simulink model "V2x_TC_TrafficLights_cs"  (Simulink 7.6)
settings: Solver=ode5  SolverMode=SingleTasking  StartTime=0.0  StopTime=38  FixedStep=1/100  RelTol=1e-3
PreLoadFcn:
  | check_NUTS_defined
  | 
  | assignin('base',['model_closed_' bdroot],false);addpath('../includes_V2x');
InitFcn:
  | PreScanExperimentInitFcn(bdroot)
StopFcn:
  | PreScanExperimentStopFcn(bdroot);
  | 
undefined symbols (not defined in the file or in its folder): acceleration, actorID, actorType, DiscIntNeverNeededParam, GPS_alt, GPS_lat, GPS_long, intmax, lightBarInUse, messageID, Newton, publicActorType, Raphson, rotZ, sigbuilder_block, sireneInUse, trafficLightPriority, turnAdvice, velocity, x_ini_VRML, y_ini_VRML, yawRate, z_ini_VRML
libraries: simulink x172, PreScanSimulinkLibrary x20, PreScanUsefulBlocks x11, simulink_extras x9, simulink_need_slupdate x1
(part 2 of 27)

== system V2x_TC_TrafficLights_cs/Antenna_Rx_1 ==
  Selector : Selector  IndexMode=Zero-based  IndexOptions=Starting index (dialog)  Indices=PortIndex  OutputSizes=PortWidth(1)
  U : Outport
  wires:
  Selector/1 -> U/1
  [SimCoreSensors] -> Selector/1

== system V2x_TC_TrafficLights_cs/Antenna_Rx_2 ==
  Selector : Selector  IndexMode=Zero-based  IndexOptions=Starting index (dialog)  Indices=PortIndex  OutputSizes=PortWidth(1)
  U : Outport
  wires:
  Selector/1 -> U/1
  [SimCoreSensors] -> Selector/1

== system V2x_TC_TrafficLights_cs/Antenna_Rx_3 ==
  Selector : Selector  IndexMode=Zero-based  IndexOptions=Starting index (dialog)  Indices=PortIndex  OutputSizes=PortWidth(1)
  U : Outport
  wires:
  Selector/1 -> U/1
  [SimCoreSensors] -> Selector/1

== system V2x_TC_TrafficLights_cs/Antenna_Rx_4 ==
  Selector : Selector  IndexMode=Zero-based  IndexOptions=Starting index (dialog)  Indices=PortIndex  OutputSizes=PortWidth(1)
  U : Outport
  wires:
  Selector/1 -> U/1
  [SimCoreSensors] -> Selector/1

== system V2x_TC_TrafficLights_cs/Antenna_Rx_5 ==
  Selector : Selector  IndexMode=Zero-based  IndexOptions=Starting index (dialog)  Indices=PortIndex  OutputSizes=PortWidth(1)
  U : Outport
  wires:
  Selector/1 -> U/1
  [SimCoreSensors] -> Selector/1

== system V2x_TC_TrafficLights_cs/Antenna_Tx_1 ==
  Y : Inport
  Assignment : Assignment  IndexMode=Zero-based  SampleTime=TimeStep  IndexOptions=Starting index (dialog)  Indices=PortIndex  OutputSizes=[1 1]
  Data Store Read : DataStoreRead  DataStoreName=SimCoreActuators  SampleTime=TimeStep
  Data Store Write : DataStoreWrite  DataStoreName=SimCoreActuators
  wires:
  Assignment/1 -> Data Store Write/1
  Data Store Read/1 -> Assignment/1
  Y/1 -> Assignment/2

== system V2x_TC_TrafficLights_cs/Antenna_Tx_10 ==
  Y : Inport
  Assignment : Assignment  IndexMode=Zero-based  SampleTime=TimeStep  IndexOptions=Starting index (dialog)  Indices=PortIndex  OutputSizes=[1 1]
  Data Store Read : DataStoreRead  DataStoreName=SimCoreActuators  SampleTime=TimeStep
  Data Store Write : DataStoreWrite  DataStoreName=SimCoreActuators
  wires:
  Assignment/1 -> Data Store Write/1
  Data Store Read/1 -> Assignment/1
  Y/1 -> Assignment/2

== system V2x_TC_TrafficLights_cs/Antenna_Tx_11 ==
  Y : Inport
  Assignment : Assignment  IndexMode=Zero-based  SampleTime=TimeStep  IndexOptions=Starting index (dialog)  Indices=PortIndex  OutputSizes=[1 1]
  Data Store Read : DataStoreRead  DataStoreName=SimCoreActuators  SampleTime=TimeStep
  Data Store Write : DataStoreWrite  DataStoreName=SimCoreActuators
  wires:
  Assignment/1 -> Data Store Write/1
  Data Store Read/1 -> Assignment/1
  Y/1 -> Assignment/2

== system V2x_TC_TrafficLights_cs/Antenna_Tx_12 ==
  Y : Inport
  Assignment : Assignment  IndexMode=Zero-based  SampleTime=TimeStep  IndexOptions=Starting index (dialog)  Indices=PortIndex  OutputSizes=[1 1]
  Data Store Read : DataStoreRead  DataStoreName=SimCoreActuators  SampleTime=TimeStep
  Data Store Write : DataStoreWrite  DataStoreName=SimCoreActuators
  wires:
  Assignment/1 -> Data Store Write/1
  Data Store Read/1 -> Assignment/1
  Y/1 -> Assignment/2

== system V2x_TC_TrafficLights_cs/Antenna_Tx_13 ==
  Y : Inport
  Assignment : Assignment  IndexMode=Zero-based  SampleTime=TimeStep  IndexOptions=Starting index (dialog)  Indices=PortIndex  OutputSizes=[1 1]
  Data Store Read : DataStoreRead  DataStoreName=SimCoreActuators  SampleTime=TimeStep
  Data Store Write : DataStoreWrite  DataStoreName=SimCoreActuators
  wires:
  Assignment/1 -> Data Store Write/1
  Data Store Read/1 -> Assignment/1
  Y/1 -> Assignment/2

== system V2x_TC_TrafficLights_cs/Antenna_Tx_14 ==
  Y : Inport
  Assignment : Assignment  IndexMode=Zero-based  SampleTime=TimeStep  IndexOptions=Starting index (dialog)  Indices=PortIndex  OutputSizes=[1 1]
  Data Store Read : DataStoreRead  DataStoreName=SimCoreActuators  SampleTime=TimeStep
  Data Store Write : DataStoreWrite  DataStoreName=SimCoreActuators
  wires:
  Assignment/1 -> Data Store Write/1
  Data Store Read/1 -> Assignment/1
  Y/1 -> Assignment/2

== system V2x_TC_TrafficLights_cs/Antenna_Tx_15 ==
  Y : Inport
  Assignment : Assignment  IndexMode=Zero-based  SampleTime=TimeStep  IndexOptions=Starting index (dialog)  Indices=PortIndex  OutputSizes=[1 1]
  Data Store Read : DataStoreRead  DataStoreName=SimCoreActuators  SampleTime=TimeStep
  Data Store Write : DataStoreWrite  DataStoreName=SimCoreActuators
  wires:
  Assignment/1 -> Data Store Write/1
  Data Store Read/1 -> Assignment/1
  Y/1 -> Assignment/2

== system V2x_TC_TrafficLights_cs/Antenna_Tx_16 ==
  Y : Inport
  Assignment : Assignment  IndexMode=Zero-based  SampleTime=TimeStep  IndexOptions=Starting index (dialog)  Indices=PortIndex  OutputSizes=[1 1]
  Data Store Read : DataStoreRead  DataStoreName=SimCoreActuators  SampleTime=TimeStep
  Data Store Write : DataStoreWrite  DataStoreName=SimCoreActuators
  wires:
  Assignment/1 -> Data Store Write/1
  Data Store Read/1 -> Assignment/1
  Y/1 -> Assignment/2

== system V2x_TC_TrafficLights_cs/Antenna_Tx_17 ==
  Y : Inport
  Assignment : Assignment  IndexMode=Zero-based  SampleTime=TimeStep  IndexOptions=Starting index (dialog)  Indices=PortIndex  OutputSizes=[1 1]
  Data Store Read : DataStoreRead  DataStoreName=SimCoreActuators  SampleTime=TimeStep
  Data Store Write : DataStoreWrite  DataStoreName=SimCoreActuators
  wires:
  Assignment/1 -> Data Store Write/1
  Data Store Read/1 -> Assignment/1
  Y/1 -> Assignment/2

== system V2x_TC_TrafficLights_cs/Antenna_Tx_18 ==
  Y : Inport
  Assignment : Assignment  IndexMode=Zero-based  SampleTime=TimeStep  IndexOptions=Starting index (dialog)  Indices=PortIndex  OutputSizes=[1 1]
  Data Store Read : DataStoreRead  DataStoreName=SimCoreActuators  SampleTime=TimeStep
  Data Store Write : DataStoreWrite  DataStoreName=SimCoreActuators
  wires:
  Assignment/1 -> Data Store Write/1
  Data Store Read/1 -> Assignment/1
  Y/1 -> Assignment/2

== system V2x_TC_TrafficLights_cs/Antenna_Tx_19 ==
  Y : Inport
  Assignment : Assignment  IndexMode=Zero-based  SampleTime=TimeStep  IndexOptions=Starting index (dialog)  Indices=PortIndex  OutputSizes=[1 1]
  Data Store Read : DataStoreRead  DataStoreName=SimCoreActuators  SampleTime=TimeStep
  Data Store Write : DataStoreWrite  DataStoreName=SimCoreActuators
  wires:
  Assignment/1 -> Data Store Write/1
  Data Store Read/1 -> Assignment/1
  Y/1 -> Assignment/2

== system V2x_TC_TrafficLights_cs/Antenna_Tx_2 ==
  Y : Inport
  Assignment : Assignment  IndexMode=Zero-based  SampleTime=TimeStep  IndexOptions=Starting index (dialog)  Indices=PortIndex  OutputSizes=[1 1]
  Data Store Read : DataStoreRead  DataStoreName=SimCoreActuators  SampleTime=TimeStep
  Data Store Write : DataStoreWrite  DataStoreName=SimCoreActuators
  wires:
  Assignment/1 -> Data Store Write/1
  Data Store Read/1 -> Assignment/1
  Y/1 -> Assignment/2

== system V2x_TC_TrafficLights_cs/Antenna_Tx_20 ==
  Y : Inport
  Assignment : Assignment  IndexMode=Zero-based  SampleTime=TimeStep  IndexOptions=Starting index (dialog)  Indices=PortIndex  OutputSizes=[1 1]
  Data Store Read : DataStoreRead  DataStoreName=SimCoreActuators  SampleTime=TimeStep
  Data Store Write : DataStoreWrite  DataStoreName=SimCoreActuators
  wires:
  Assignment/1 -> Data Store Write/1
  Data Store Read/1 -> Assignment/1
  Y/1 -> Assignment/2

== system V2x_TC_TrafficLights_cs/Antenna_Tx_21 ==
  Y : Inport
  Assignment : Assignment  IndexMode=Zero-based  SampleTime=TimeStep  IndexOptions=Starting index (dialog)  Indices=PortIndex  OutputSizes=[1 1]
  Data Store Read : DataStoreRead  DataStoreName=SimCoreActuators  SampleTime=TimeStep
  Data Store Write : DataStoreWrite  DataStoreName=SimCoreActuators
  wires:
  Assignment/1 -> Data Store Write/1
  Data Store Read/1 -> Assignment/1
  Y/1 -> Assignment/2

== system V2x_TC_TrafficLights_cs/Antenna_Tx_22 ==
  Y : Inport
  Assignment : Assignment  IndexMode=Zero-based  SampleTime=TimeStep  IndexOptions=Starting index (dialog)  Indices=PortIndex  OutputSizes=[1 1]
  Data Store Read : DataStoreRead  DataStoreName=SimCoreActuators  SampleTime=TimeStep
  Data Store Write : DataStoreWrite  DataStoreName=SimCoreActuators
  wires:
  Assignment/1 -> Data Store Write/1
  Data Store Read/1 -> Assignment/1
  Y/1 -> Assignment/2

== system V2x_TC_TrafficLights_cs/Antenna_Tx_23 ==
  Y : Inport
  Assignment : Assignment  IndexMode=Zero-based  SampleTime=TimeStep  IndexOptions=Starting index (dialog)  Indices=PortIndex  OutputSizes=[1 1]
  Data Store Read : DataStoreRead  DataStoreName=SimCoreActuators  SampleTime=TimeStep
  Data Store Write : DataStoreWrite  DataStoreName=SimCoreActuators
  wires:
  Assignment/1 -> Data Store Write/1
  Data Store Read/1 -> Assignment/1
  Y/1 -> Assignment/2

== system V2x_TC_TrafficLights_cs/Antenna_Tx_3 ==
  Y : Inport
  Assignment : Assignment  IndexMode=Zero-based  SampleTime=TimeStep  IndexOptions=Starting index (dialog)  Indices=PortIndex  OutputSizes=[1 1]
  Data Store Read : DataStoreRead  DataStoreName=SimCoreActuators  SampleTime=TimeStep
  Data Store Write : DataStoreWrite  DataStoreName=SimCoreActuators
  wires:
  Assignment/1 -> Data Store Write/1
  Data Store Read/1 -> Assignment/1
  Y/1 -> Assignment/2

== system V2x_TC_TrafficLights_cs/Antenna_Tx_4 ==
  Y : Inport
  Assignment : Assignment  IndexMode=Zero-based  SampleTime=TimeStep  IndexOptions=Starting index (dialog)  Indices=PortIndex  OutputSizes=[1 1]
  Data Store Read : DataStoreRead  DataStoreName=SimCoreActuators  SampleTime=TimeStep
  Data Store Write : DataStoreWrite  DataStoreName=SimCoreActuators
  wires:
  Assignment/1 -> Data Store Write/1
  Data Store Read/1 -> Assignment/1
  Y/1 -> Assignment/2

== system V2x_TC_TrafficLights_cs/Antenna_Tx_5 ==
  Y : Inport
  Assignment : Assignment  IndexMode=Zero-based  SampleTime=TimeStep  IndexOptions=Starting index (dialog)  Indices=PortIndex  OutputSizes=[1 1]
  Data Store Read : DataStoreRead  DataStoreName=SimCoreActuators  SampleTime=TimeStep
  Data Store Write : DataStoreWrite  DataStoreName=SimCoreActuators
  wires:
  Assignment/1 -> Data Store Write/1
  Data Store Read/1 -> Assignment/1
  Y/1 -> Assignment/2

== system V2x_TC_TrafficLights_cs/Antenna_Tx_6 ==
  Y : Inport
  Assignment : Assignment  IndexMode=Zero-based  SampleTime=TimeStep  IndexOptions=Starting index (dialog)  Indices=PortIndex  OutputSizes=[1 1]
  Data Store Read : DataStoreRead  DataStoreName=SimCoreActuators  SampleTime=TimeStep
  Data Store Write : DataStoreWrite  DataStoreName=SimCoreActuators
  wires:
  Assignment/1 -> Data Store Write/1
  Data Store Read/1 -> Assignment/1
  Y/1 -> Assignment/2

== system V2x_TC_TrafficLights_cs/Antenna_Tx_7 ==
  Y : Inport
  Assignment : Assignment  IndexMode=Zero-based  SampleTime=TimeStep  IndexOptions=Starting index (dialog)  Indices=PortIndex  OutputSizes=[1 1]
  Data Store Read : DataStoreRead  DataStoreName=SimCoreActuators  SampleTime=TimeStep
  Data Store Write : DataStoreWrite  DataStoreName=SimCoreActuators
  wires:
  Assignment/1 -> Data Store Write/1
  Data Store Read/1 -> Assignment/1
  Y/1 -> Assignment/2

== system V2x_TC_TrafficLights_cs/Antenna_Tx_8 ==
  Y : Inport
  Assignment : Assignment  IndexMode=Zero-based  SampleTime=TimeStep  IndexOptions=Starting index (dialog)  Indices=PortIndex  OutputSizes=[1 1]
  Data Store Read : DataStoreRead  DataStoreName=SimCoreActuators  SampleTime=TimeStep
  Data Store Write : DataStoreWrite  DataStoreName=SimCoreActuators
  wires:
  Assignment/1 -> Data Store Write/1
  Data Store Read/1 -> Assignment/1
  Y/1 -> Assignment/2

== system V2x_TC_TrafficLights_cs/Antenna_Tx_9 ==
  Y : Inport
  Assignment : Assignment  IndexMode=Zero-based  SampleTime=TimeStep  IndexOptions=Starting index (dialog)  Indices=PortIndex  OutputSizes=[1 1]
  Data Store Read : DataStoreRead  DataStoreName=SimCoreActuators  SampleTime=TimeStep
  Data Store Write : DataStoreWrite  DataStoreName=SimCoreActuators
  wires:
  Assignment/1 -> Data Store Write/1
  Data Store Read/1 -> Assignment/1
  Y/1 -> Assignment/2

== system V2x_TC_TrafficLights_cs/Audi_A8_1 ==
  SELF_Audi_A8_1 : Inport  Tag=SELFInport
  Antenna_Rx_1 : Inport  Tag=AntennaRInport  Port=2
  ANIMATION PORTS Tuning : Subsystem
  Antenna_Rx_1_BusSelector : BusSelector  Tag=BusAntennaR  OutputSignals=warning,messageID,actorID,actorType,publicActorType,lightBarInUse,sireneInUse,velocity,acceleration,yawRate,turnAdvice,trafficLightPriority,GPS_lat,GPS_long,GPS_alt,rotZ
  Antenna_Rx_1_Demux : Subsystem  Tag=DemuxAntennaR  mask(PreScan Demux Block:){Channel=1}
      settings: DeleteFcn=PreScanBlockDeleteFcn;
      mask Display:
      | fprintf('Channel: %d',Channel)
      | port_label('output', 1, 'Vehicle_ID')
      | port_label('output', 2, 'GPS Pos Lat [deg]')
      | port_label('output', 3, 'GPS Pos Long [deg]')
      | port_label('output', 4, 'GPS Pos Alt [m]')
      | port_label('output', 5, 'Velocity [m/s]')
      | port_label('output', 6, 'Acceleration [m/s2]')
      | port_label('output', 7, 'Heading [deg]')
      | port_label('output', 8, 'Warning signal ID')
      | port_label('output', 9, 'Maneuvre ID')
      | port_label('output', 10, 'Vehicle Type')
      | port_label('output', 11, 'Braking Capacity')
      | port_label('output', 12, 'Vehicle Size')
      | port_label('output', 13, 'Spare')
  Antenna_Tx_1_BusAssignment : BusAssignment  Tag=BusAntennaT  AssignedSignals=warning,messageID,actorID,actorType,publicActorType,lightBarInUse,sireneInUse,velocity,acceleration,yawRate,turnAdvice,trafficLightPriority,GPS_lat,GPS_long,GPS_alt,rotZ
  Antenna_Tx_1_Mux : Subsystem  Tag=MuxAntennaT  mask(PreScan Mux Block:){Channel=1, TimeStep=1/100}
      mask prompts: TimeStep "Time Step [s]"
      settings: DeleteFcn=PreScanBlockDeleteFcn;
      mask Display:
      | fprintf('Channel: %d',Channel)
      | port_label('input', 1, 'Warning signal ID')
      | port_label('input', 2, 'Maneuvre ID')
      | port_label('input', 3, 'Vehicle Type')
      | port_label('input', 4, 'Braking Capacity')
      | port_label('input', 5, 'Vehicle Size')
      | port_label('input', 6, 'spare')
  Constant : Constant  Value=0
  Constant1 : Constant  Value=15
  Dynamics_Simple : Subsystem  Tag=Simple_Vehicle_Dynamics  mask(PreScan Block: Simple Dynamics){Migrate=off, TimeStep=1/100, ComputationMode=Continuous_Time}
      mask prompts: Migrate "Migrate. During migration this block will be replaced by a newer one."; TimeStep "Auto assigned: Time Step [s]"; ComputationMode "Computation mode:"
      mask help:
      | The Simple Dynamics Model is selected in the GUI to simulate vehicle dynamics behavior.
      |
      | The Simple Dynamics Model is completely open to the user. The parameters of this model can be set in the Simple Dynamics dialog in the GUI.
      |
      | For more information, please click Help.
      mask init:
      | p = SimpleDyn_pars;
      settings: DeleteFcn=PreScanBlockDeleteFcn;
      mask Help: eval('openHelp(220)')
      mask Display:
      | image(imread('SimpleDyn.png'))
      | port_label('input', 1, '{\delta}_S_t_ _e_e_r [deg]','texmode','on')
      | port_label('input', 2, 'Throttle [%]')
      | port_label('input', 3, 'Brake Pres [bar]')
      | port_label('input', 4, 'V_0 [ms-1]','texmode','on')
      | port_label('input', 5, 'Auto=[0]/Manual=[1]')
      | port_label('input', 6, 'Auto_GearShift')
      | port_label('input', 7, 'Manual_GearShift')
      | port_label('output', 1, 'State')
      | port_label('output', 2, 'Vx [ms-1]')
      | port_label('output', 3, 'Perf Params')
  Ground_{1..18} (18 alike) : Ground
  Mux : Mux  Inputs=3
  Mux1 : Mux  Inputs=3
  PathFollower_1 : Subsystem  Tag=pathFollower  mask(PreScan Block: Path Follower){Migrate=on, FileName='Trajectory_1.mat', TunableSteeringGain=1, XShifting_wrt_COG=0}
      mask prompts: Migrate "Migrate. During migration this block will be replaced by a newer one."; FileName "Trajectory Name"; TunableSteeringGain "TunableSteeringGain [-]"; XShifting_wrt_COG "XShifting_wrt_COG [m]"
      mask help:
      | The Path Follower Model is selected in the GUI to simulate driver behavior.
      |
      | For more information, please click Help.
      mask init:
      | load(['trajectories/',eval(FileName)])
      | traject=trajectory';
      | 
      | Traj_x=traject(:,2);
      | Traj_y=traject(:,3);
      | Traj_z=traject(:,4);
      | Traj_vx=traject(:,8);
      | Traj_vy=traject(:,9);
      | Traj_vz=traject(:,10);
      mask Help: eval('openHelp(232)')
  RA_distance : Scope
      settings: YMin=2.5  YMax=55  SaveName=ScopeData7
  RA_warning : Scope
      settings: YMin=-1  YMax=1  SaveName=ScopeData6
  Rate Transition1 : RateTransition  Integrity=off
  Rate Transition2 : RateTransition  Integrity=off
  Rx_Noise : Subsystem
  SELF_Demux : Subsystem  Tag=DemuxSELF
      settings: DeleteFcn=PreScanBlockDeleteFcn;
      mask Type: PreScan Demux Block:
      mask Display:
      | port_label('output',1,'x [m]')
      | port_label('output',2,'y [m]')
      | port_label('output',3,'z [m]','texmode','on')
      | port_label('output',4,'Rotx [deg]','texmode','on')
      | port_label('output',5,'Roty [deg]','texmode','on')
      | port_label('output',6,'Rotz [deg]','texmode','on')
      | port_label('output',7,'GPS lat [deg]','texmode','on')
      | port_label('output',8,'GPS long [deg]','texmode','on')
      | port_label('output',9,'GPS alt [m]','texmode','on')
      | port_label('output',10,'Velocity [m/s]')
      | port_label('output',11,'Heading [deg]')
      | port_label('output',12,'Yaw rate [deg/s]')
  Subsystem : Subsystem
  Subsystem1 : Subsystem
  Terminator_{1..23} (23 alike) : Terminator
  Traffic Controller : Subsystem
  Trajectories : Subsystem  Tag=Trajectories
      settings: DeleteFcn=PreScanBlockDeleteFcn;
      mask Type: PreScan Source Block: Trajectories
      mask Description:
      | The Trajectory block contains the trajectories defined in the PreScan Experiment Editor. The output of the trajectory block is the (vehicle) State.
      | 
      | For more information, please click Help.
      mask Help: eval('openHelp(312)')
      mask Display: image(imread('Trajectory.png'));
  Unit Delay : UnitDelay  SampleTime=-1
  Unit Delay1 : UnitDelay  SampleTime=-1
  V2x_TC_GUI : Subsystem
  VISULightSource_Mux : Subsystem  Tag=MuxLightSource
      settings: DeleteFcn=PreScanBlockDeleteFcn;
      mask Type: PreScan Mux Block:
  VISUSteer_Mux : Subsystem  Tag=MuxSteer
      settings: DeleteFcn=PreScanBlockDeleteFcn;
      mask Type: PreScan Mux Block:
  VISUWheels_Mux : Subsystem  Tag=MuxWheels  mask(PreScan Mux Block:){TimeStep=1/100}
      mask prompts: TimeStep "Auto Assigned: Time Step [s]"
      settings: DeleteFcn=PreScanBlockDeleteFcn;
  des vel : Subsystem
  "intersection warning {left,straight,right}" : Scope
      settings: YMin=-0.1  YMax=1.1  SaveName=ScopeData5
  lightsMessage : Scope
      settings: SaveName=ScopeData2
  lightsPriority : Scope
      settings: SaveName=ScopeData3
  lightsWarning : Scope
      settings: SaveName=ScopeData4
  pos : Constant  Tag=pos  Value=[25.04266357421875 11.706245422363283 0]
  signsMessage : Scope
      settings: TimeRange=0.1843234764159973  YMin=900.4078101828966  YMax=905.9158957700726
  signsWarning : Scope
  vehicle CAM generator : Subsystem
  STATE_Audi_A8_1 : Outport  Tag=StateOutport
  Antenna_Tx_1 : Outport  Tag=AntennaTOutport  Port=2
  VISULightSource_Audi_A8_1 : Outport  Tag=VisuLightsOutport  Port=3  SampleTime=1/100
  VISUWheels_Audi_A8_1 : Outport  Tag=VisuWheelsOutport  Port=4  SampleTime=1/100
  VISUSteer_Audi_A8_1 : Outport  Tag=VisuSteerOutport  Port=5  SampleTime=1/100
  tag scope: State=global, perf=global
  wires:
  Constant/1 -> vehicle CAM generator/10(turnAdvice), Traffic Controller/1(hostTurnAdvice)
  Mux/1 -> "intersection warning {left,straight,right}"/1
  [perf] -> Rate Transition2/1
  Traffic Controller/1(signsWarning) -> signsWarning/1, V2x_TC_GUI/1(signsWarning)
  Traffic Controller/2(signsMessage) -> signsMessage/1, V2x_TC_GUI/2(signsMessage)
  Traffic Controller/3(lightsMessage) -> lightsMessage/1
  Traffic Controller/4(lightsPriority) -> lightsPriority/1
  Traffic Controller/5(lightsWarning) -> lightsWarning/1, V2x_TC_GUI/3(lightsWarning)
  Traffic Controller/6(warning left lane) -> Mux/1
  Traffic Controller/7(warning straight lane) -> Mux/2
  Traffic Controller/8(warning right lane) -> Mux/3
  Traffic Controller/9(RA_warning) -> RA_warning/1
  Traffic Controller/10(RA_distance) -> RA_distance/1
  Rate Transition1/1 -> ANIMATION PORTS Tuning/1(state)
  [State] -> Rate Transition1/1
  Rate Transition2/1 -> V2x_TC_GUI/4(perf)
  Rx_Noise/5(CAM_publicVehicleType) -> Terminator_16/1
  Rx_Noise/6(CAM_lightBarInUse) -> Terminator_17/1
  Rx_Noise/7(CAM_sireneInUse) -> Terminator_18/1
  Rx_Noise/9(CAM_acceleration) -> Terminator_19/1
  Rx_Noise/10(CAM_yawRate) -> Terminator_20/1
  [NMM_Noise] -> Rx_Noise/17(NoMissedMessages)
  [Tdelay] -> Rx_Noise/18(MessageDelay)
  Rx_Noise/1(CAM_warning) -> Traffic Controller/7(warning)
  Rx_Noise/2(CAM_messageID) -> Traffic Controller/8(messageID)
  Rx_Noise/3(CAM_vehicleID) -> Traffic Controller/9(actorID)
  Rx_Noise/4(CAM_vehicleType) -> Traffic Controller/10(actorType)
  Rx_Noise/8(CAM_velocity) -> Traffic Controller/11(velocity)
  Rx_Noise/11(CAM_turnAdvice) -> Traffic Controller/12(turnAdvice)
  Rx_Noise/12(CAM_trafficLightPriority) -> Traffic Controller/13(trafficLightPriority)
  Rx_Noise/13(CAM_GPSlat) -> Traffic Controller/14(GPS_lat)
  Rx_Noise/14(CAM_GPSlong) -> Traffic Controller/15(GPS_long)
  Rx_Noise/15(CAM_GPSalt) -> Traffic Controller/16(GPS_alt)
  Rx_Noise/16(CAM_rotZ) -> Traffic Controller/17(rotZ)
  [perf] -> Subsystem/1(In1)
  Subsystem/1(Out1) -> Unit Delay1/1
  pos/1 -> PathFollower_1/1(TrajOffset_x,y,Y [m,m,deg])
  SELF_Audi_A8_1/1 -> SELF_Demux/1(SELF)
  Antenna_Tx_1_Mux/2(Out2) -> [Antenna_Tx_1]
  [Antenna_Tx_1] -> Antenna_Tx_1_BusAssignment/1
  Antenna_Tx_1_Mux/1(Out1) -> Antenna_Tx_1/1
  Antenna_Rx_1_Demux/13(spare) -> Antenna_Rx_1_BusSelector/1
  Antenna_Rx_1/1 -> Antenna_Rx_1_Demux/1(AntennaR)
  VISULightSource_Mux/1(Lights) -> VISULightSource_Audi_A8_1/1
  VISUWheels_Mux/1(Wheels) -> VISUWheels_Audi_A8_1/1
  VISUSteer_Mux/1(Steer) -> VISUSteer_Audi_A8_1/1
  Dynamics_Simple/2(Vx [m s-1]) -> Terminator_23/1
  PathFollower_1/1(delta_steerwheel [deg]) -> Dynamics_Simple/1(DeltaSteer_[deg])
  PathFollower_1/2(throttle [%]) -> Dynamics_Simple/2(Throttle)
  PathFollower_1/3(brake pressure [bar]) -> Dynamics_Simple/3(Brake)
  PathFollower_1/4(v0 [m/s]) -> Dynamics_Simple/4(Initial_Vx_[ms-1])
  PathFollower_1/5(Auto_GearShift) -> Dynamics_Simple/6(Auto_GearShift)
  Dynamics_Simple/1(State) -> STATE_Audi_A8_1/1, [State]
  SELF_Demux/5(roty [deg]) -> Terminator_15/1
  SELF_Demux/4(rotx [deg]) -> Terminator_14/1
  PathFollower_1/7(Actor/Traj_angle [deg]) -> Terminator_21/1
  PathFollower_1/6(Actor/Traj_distance [m]) -> Terminator_22/1
  vehicle CAM generator/16(CAM_rotZ) -> Antenna_Tx_1_BusAssignment/17
  vehicle CAM generator/15(CAM_GPSalt) -> Antenna_Tx_1_BusAssignment/16
  vehicle CAM generator/14(CAM_GPSlong) -> Antenna_Tx_1_BusAssignment/15
  vehicle CAM generator/13(CAM_GPSlat) -> Antenna_Tx_1_BusAssignment/14
  vehicle CAM generator/12(CAM_trafficLightPriority) -> Antenna_Tx_1_BusAssignment/13
  vehicle CAM generator/11(CAM_turnAdvice) -> Antenna_Tx_1_BusAssignment/12
  vehicle CAM generator/8(CAM_velocity) -> Antenna_Tx_1_BusAssignment/9
  Antenna_Rx_1_BusSelector/16 -> Rx_Noise/16(CAM_rotZ_in)  "<rotZ>"
  Antenna_Rx_1_BusSelector/15 -> Rx_Noise/15(CAM_GPSalt_in)  "<GPS_alt>"
  Antenna_Rx_1_BusSelector/14 -> Rx_Noise/14(CAM_GPSlong_in)  "<GPS_long>"
  Antenna_Rx_1_BusSelector/13 -> Rx_Noise/13(CAM_GPSlat_in)  "<GPS_lat>"
  Antenna_Rx_1_BusSelector/12 -> Rx_Noise/12(CAM_trafficLightPriority_in)  "<trafficLightPriority>"
  Antenna_Rx_1_BusSelector/11 -> Rx_Noise/11(CAM_turnAdvice_in)  "<turnAdvice>"
  Antenna_Rx_1_BusSelector/10 -> Rx_Noise/10(CAM_yawRate_in)  "<yawRate>"
  Antenna_Rx_1_BusSelector/9 -> Rx_Noise/9(CAM_acceleration_in)  "<acceleration>"
  Antenna_Rx_1_BusSelector/8 -> Rx_Noise/8(CAM_velocity_in)  "<velocity>"
  Antenna_Rx_1_BusSelector/7 -> Rx_Noise/7(CAM_sireneInUse_in)  "<sireneInUse>"
  Antenna_Rx_1_BusSelector/6 -> Rx_Noise/6(CAM_lightBarInUse_in)  "<lightBarInUse>"
  Antenna_Rx_1_BusSelector/5 -> Rx_Noise/5(CAM_publicVehicleType_in)  "<publicActorType>"
  Antenna_Rx_1_BusSelector/4 -> Rx_Noise/4(CAM_vehicleType_in)  "<actorType>"
  Antenna_Rx_1_BusSelector/3 -> Rx_Noise/3(CAM_vehicleID_in)  "<actorID>"
  Antenna_Rx_1_BusSelector/2 -> Rx_Noise/2(CAM_messageID_in)  "<messageID>"
  Antenna_Rx_1_BusSelector/1 -> Rx_Noise/1(CAM_warning_in)  "<warning>"
  Constant1/1 -> PathFollower_1/4(Initial Velocity [m/s])
  des vel/1(vel) -> PathFollower_1/5(Desired Velocity [m/s])
  Trajectories/1(State) -> des vel/1(state)
  Mux1/1 -> PathFollower_1/3(position x,y,Y [m,m,deg])
  ANIMATION PORTS Tuning/6(zDisp RR [m]) -> VISUWheels_Mux/7(zDisp RR [m])
  ANIMATION PORTS Tuning/5(zDisp RL [m]) -> VISUWheels_Mux/6(zDisp RL [m])
  ANIMATION PORTS Tuning/4(zDisp FR [m]) -> VISUWheels_Mux/5(zDisp FR [m])
  ANIMATION PORTS Tuning/3(zDisp FL [m]) -> VISUWheels_Mux/4(zDisp FL [m])
  ANIMATION PORTS Tuning/1(Steer Angle Front [deg]) -> VISUWheels_Mux/1(Steer Angle Front [deg])
  ANIMATION PORTS Tuning/2(Velocity [m/s]) -> VISUWheels_Mux/3(Velocity [m/s])
  ANIMATION PORTS Tuning/7(Column [deg]) -> VISUSteer_Mux/1(Column [deg])
  ANIMATION PORTS Tuning/8(Steer [deg]) -> VISUSteer_Mux/2(Steer [deg])
  SELF_Demux/12(yaw rate [deg/s]) -> vehicle CAM generator/9(yawRate)
  SELF_Demux/10(velocity [m/s]) -> PathFollower_1/6(velocity [m/s]), vehicle CAM generator/8(velocity [m/s]), Traffic Controller/6(hostVelocity)
  SELF_Demux/9(GPS alt [m]) -> vehicle CAM generator/7(GPS_alt), Traffic Controller/5(host GPS alt [m])
  SELF_Demux/8(GPS long [deg]) -> vehicle CAM generator/6(GPS_long), Traffic Controller/4(host GPS long [deg])
  SELF_Demux/7(GPS lat [deg]) -> vehicle CAM generator/5(GPS_lat), Traffic Controller/3(host GPS lat [deg])
  SELF_Demux/6(rotz [deg]) -> vehicle CAM generator/4(rotZ [deg]), Mux1/3, Traffic Controller/2(hostRotZ)
  SELF_Demux/3(z [m]) -> vehicle CAM generator/3(z [m])
  SELF_Demux/2(y [m]) -> vehicle CAM generator/2(y [m]), Mux1/2
  SELF_Demux/1(x [m]) -> vehicle CAM generator/1(x [m]), Mux1/1
  vehicle CAM generator/1(CAM_warning) -> Antenna_Tx_1_BusAssignment/2
  vehicle CAM generator/2(CAM_messageID) -> Antenna_Tx_1_BusAssignment/3
  vehicle CAM generator/3(CAM_actorID) -> Antenna_Tx_1_BusAssignment/4
  vehicle CAM generator/4(CAM_actorType) -> Antenna_Tx_1_BusAssignment/5
  vehicle CAM generator/5(CAM_publicActorType) -> Antenna_Tx_1_BusAssignment/6
  vehicle CAM generator/6(CAM_lightBarInUse) -> Antenna_Tx_1_BusAssignment/7
  vehicle CAM generator/7(CAM_sireneInUse) -> Antenna_Tx_1_BusAssignment/8
  vehicle CAM generator/9(CAM_acceleration) -> Antenna_Tx_1_BusAssignment/10
  vehicle CAM generator/10(CAM_yawRate) -> Antenna_Tx_1_BusAssignment/11
  Dynamics_Simple/3(Perf_Params) -> [perf]
  Subsystem1/1(Left) -> VISULightSource_Mux/1(IndicatorLightsLeft)
  Ground_18/1 -> VISULightSource_Mux/2(IndicatorLightsRight)
  Unit Delay/1 -> Antenna_Tx_1_Mux/6(spare)
  Unit Delay1/1 -> VISULightSource_Mux/11(BrakeLightLeft), VISULightSource_Mux/12(BrakeLightRight)
  Antenna_Tx_1_BusAssignment/1 -> Unit Delay/1
  Antenna_Rx_1_Demux/1(Vehicle_ID) -> Terminator_1/1
  Antenna_Rx_1_Demux/2(GPS_pos_lat_[deg]) -> Terminator_2/1
  Antenna_Rx_1_Demux/3(GPS_pos_long_[deg]) -> Terminator_3/1
  Antenna_Rx_1_Demux/4(GPS_pos_alt_[m]) -> Terminator_4/1
  Antenna_Rx_1_Demux/5(Velocity_[m/s]) -> Terminator_5/1
  Antenna_Rx_1_Demux/6(Acceleration_[m/s2]) -> Terminator_6/1
  Antenna_Rx_1_Demux/7(Heading [deg]) -> Terminator_7/1
  Antenna_Rx_1_Demux/8(warning_signal_id) -> Terminator_8/1
  Antenna_Rx_1_Demux/9(maneuvre_id) -> Terminator_9/1
  Antenna_Rx_1_Demux/10(vehicle_type) -> Terminator_10/1
  Antenna_Rx_1_Demux/11(brakingcapacity) -> Terminator_11/1
  Antenna_Rx_1_Demux/12(vehicle_size) -> Terminator_12/1
  Ground_1/1 -> Antenna_Tx_1_Mux/1(warning_signal_id)
  Ground_2/1 -> Antenna_Tx_1_Mux/2(maneuvre_id)
  Ground_3/1 -> Antenna_Tx_1_Mux/3(vehicle_type)
  Ground_4/1 -> Antenna_Tx_1_Mux/4(brakingcapacity)
  Ground_5/1 -> Antenna_Tx_1_Mux/5(vehicle_size)
  Ground_6/1 -> Dynamics_Simple/5(AutoManual_OnOff)
  Ground_7/1 -> Dynamics_Simple/7(Manual_GearShift)
  Ground_8/1 -> PathFollower_1/2(ActorOffset_Left(+) / Right(-) [m])
  SELF_Demux/11(heading [deg]) -> Terminator_13/1
  Ground_9/1 -> VISULightSource_Mux/3(FogLightRearLeft)
  Ground_10/1 -> VISULightSource_Mux/4(FogLightRearRight)
  Ground_11/1 -> VISULightSource_Mux/5(FogLightFrontLeft)
  Ground_12/1 -> VISULightSource_Mux/6(FogLightFrontRight)
  Ground_13/1 -> VISULightSource_Mux/7(MainLightRearLeft)
  Ground_14/1 -> VISULightSource_Mux/8(MainLightRearRight)
  Ground_15/1 -> VISULightSource_Mux/9(MainLightFrontLeft)
  Ground_16/1 -> VISULightSource_Mux/10(MainLightFrontRight)
  Ground_17/1 -> VISUWheels_Mux/2(Steer Angle Rear [deg])

== system V2x_TC_TrafficLights_cs/BMW_Z3_1 ==
  SELF_BMW_Z3_1 : Inport  Tag=SELFInport
  Antenna_Rx_4 : Inport  Tag=AntennaRInport  Port=2
  Antenna_Rx_4_BusSelector : BusSelector  Tag=BusAntennaR  OutputSignals=warning,messageID,actorID,actorType,publicActorType,lightBarInUse,sireneInUse,velocity,acceleration,yawRate
  Antenna_Rx_4_Demux : Subsystem  Tag=DemuxAntennaR  mask(PreScan Demux Block:){Channel=1}
      settings: DeleteFcn=PreScanBlockDeleteFcn;
      mask Display:
      | fprintf('Channel: %d',Channel)
      | port_label('output', 1, 'Vehicle_ID')
      | port_label('output', 2, 'GPS Pos Lat [deg]')
      | port_label('output', 3, 'GPS Pos Long [deg]')
      | port_label('output', 4, 'GPS Pos Alt [m]')
      | port_label('output', 5, 'Velocity [m/s]')
      | port_label('output', 6, 'Acceleration [m/s2]')
      | port_label('output', 7, 'Heading [deg]')
      | port_label('output', 8, 'Warning signal ID')
      | port_label('output', 9, 'Maneuvre ID')
      | port_label('output', 10, 'Vehicle Type')
      | port_label('output', 11, 'Braking Capacity')
      | port_label('output', 12, 'Vehicle Size')
      | port_label('output', 13, 'Spare')
  Antenna_Tx_22_BusAssignment : BusAssignment  Tag=BusAntennaT  AssignedSignals=warning,messageID,actorID,actorType,publicActorType,lightBarInUse,sireneInUse,velocity,acceleration,yawRate,turnAdvice,trafficLightPriority,GPS_lat,GPS_long,GPS_alt,rotZ
  Antenna_Tx_22_Mux : Subsystem  Tag=MuxAntennaT  mask(PreScan Mux Block:){Channel=1, TimeStep=1/100}
      mask prompts: TimeStep "Time Step [s]"
      settings: DeleteFcn=PreScanBlockDeleteFcn;
      mask Display:
      | fprintf('Channel: %d',Channel)
      | port_label('input', 1, 'Warning signal ID')
      | port_label('input', 2, 'Maneuvre ID')
      | port_label('input', 3, 'Vehicle Type')
      | port_label('input', 4, 'Braking Capacity')
      | port_label('input', 5, 'Vehicle Size')
      | port_label('input', 6, 'spare')
  Constant : Constant  Value=0
  Dynamics_Empty : Subsystem  Tag=Dynamics_Empty
      mask Display: port_label('output', 1, 'State');text(0,0,' Empty\n')
  Ground_{1..5} (5 alike) : Ground
  SELF_Demux : Subsystem  Tag=DemuxSELF
      settings: DeleteFcn=PreScanBlockDeleteFcn;
      mask Type: PreScan Demux Block:
      mask Display:
      | port_label('output',1,'x [m]')
      | port_label('output',2,'y [m]')
      | port_label('output',3,'z [m]','texmode','on')
      | port_label('output',4,'Rotx [deg]','texmode','on')
      | port_label('output',5,'Roty [deg]','texmode','on')
      | port_label('output',6,'Rotz [deg]','texmode','on')
      | port_label('output',7,'GPS lat [deg]','texmode','on')
      | port_label('output',8,'GPS long [deg]','texmode','on')
      | port_label('output',9,'GPS alt [m]','texmode','on')
      | port_label('output',10,'Velocity [m/s]')
      | port_label('output',11,'Heading [deg]')
      | port_label('output',12,'Yaw rate [deg/s]')
  Terminator_{1..15,18..27} (25 alike) : Terminator
  Unit Delay1 : UnitDelay  SampleTime=-1
  vehicle CAM generator : Subsystem
  STATE_BMW_Z3_1 : Outport  Tag=StateOutport
  Antenna_Tx_22 : Outport  Tag=AntennaTOutport  Port=2
  wires:
  Constant/1 -> vehicle CAM generator/10(turnAdvice)
  [GPS_Noise] -> vehicle CAM generator/11(GPS_Noise_src)
  [MUR_Noise] -> vehicle CAM generator/12(MessageUpdateRate)
  SELF_BMW_Z3_1/1 -> SELF_Demux/1(SELF)
  Antenna_Rx_4_Demux/13(spare) -> Antenna_Rx_4_BusSelector/1
  Antenna_Rx_4/1 -> Antenna_Rx_4_Demux/1(AntennaR)
  Antenna_Tx_22_Mux/2(Out2) -> [Antenna_Tx_22]
  [Antenna_Tx_22] -> Antenna_Tx_22_BusAssignment/1
  Antenna_Tx_22_Mux/1(Out1) -> Antenna_Tx_22/1
  Dynamics_Empty/1(State) -> STATE_BMW_Z3_1/1
  Antenna_Rx_4_BusSelector/10 -> Terminator_27/1  "<yawRate>"
  Antenna_Rx_4_BusSelector/9 -> Terminator_26/1  "<acceleration>"
  Antenna_Rx_4_BusSelector/8 -> Terminator_25/1  "<velocity>"
  Antenna_Rx_4_BusSelector/7 -> Terminator_24/1  "<sireneInUse>"
  Antenna_Rx_4_BusSelector/6 -> Terminator_23/1  "<lightBarInUse>"
  Antenna_Rx_4_BusSelector/5 -> Terminator_22/1  "<publicActorType>"
  Antenna_Rx_4_BusSelector/4 -> Terminator_21/1  "<actorType>"
  Antenna_Rx_4_BusSelector/3 -> Terminator_20/1  "<actorID>"
  Antenna_Rx_4_BusSelector/2 -> Terminator_19/1  "<messageID>"
  Antenna_Rx_4_BusSelector/1 -> Terminator_18/1  "<warning>"
  vehicle CAM generator/16(CAM_rotZ) -> Antenna_Tx_22_BusAssignment/17
  vehicle CAM generator/15(CAM_GPSalt) -> Antenna_Tx_22_BusAssignment/16
  vehicle CAM generator/14(CAM_GPSlong) -> Antenna_Tx_22_BusAssignment/15
  vehicle CAM generator/13(CAM_GPSlat) -> Antenna_Tx_22_BusAssignment/14
  vehicle CAM generator/12(CAM_trafficLightPriority) -> Antenna_Tx_22_BusAssignment/13
  vehicle CAM generator/11(CAM_turnAdvice) -> Antenna_Tx_22_BusAssignment/12
  SELF_Demux/12(yaw rate [deg/s]) -> vehicle CAM generator/9(yawRate)
  SELF_Demux/10(velocity [m/s]) -> vehicle CAM generator/8(velocity [m/s])
  SELF_Demux/9(GPS alt [m]) -> vehicle CAM generator/7(GPS_alt)
  SELF_Demux/8(GPS long [deg]) -> vehicle CAM generator/6(GPS_long)
  SELF_Demux/7(GPS lat [deg]) -> vehicle CAM generator/5(GPS_lat)
  SELF_Demux/6(rotz [deg]) -> vehicle CAM generator/4(rotZ [deg])
  SELF_Demux/3(z [m]) -> vehicle CAM generator/3(z [m])
  SELF_Demux/2(y [m]) -> vehicle CAM generator/2(y [m])
  SELF_Demux/1(x [m]) -> vehicle CAM generator/1(x [m])
  vehicle CAM generator/1(CAM_warning) -> Antenna_Tx_22_BusAssignment/2
  vehicle CAM generator/2(CAM_messageID) -> Antenna_Tx_22_BusAssignment/3
  vehicle CAM generator/3(CAM_actorID) -> Antenna_Tx_22_BusAssignment/4
  vehicle CAM generator/4(CAM_actorType) -> Antenna_Tx_22_BusAssignment/5
  vehicle CAM generator/5(CAM_publicActorType) -> Antenna_Tx_22_BusAssignment/6
  vehicle CAM generator/6(CAM_lightBarInUse) -> Antenna_Tx_22_BusAssignment/7
  vehicle CAM generator/7(CAM_sireneInUse) -> Antenna_Tx_22_BusAssignment/8
  vehicle CAM generator/8(CAM_velocity) -> Antenna_Tx_22_BusAssignment/9
  vehicle CAM generator/9(CAM_acceleration) -> Antenna_Tx_22_BusAssignment/10
  vehicle CAM generator/10(CAM_yawRate) -> Antenna_Tx_22_BusAssignment/11
  Unit Delay1/1 -> Antenna_Tx_22_Mux/6(spare)
  Antenna_Tx_22_BusAssignment/1 -> Unit Delay1/1
  Antenna_Rx_4_Demux/1(Vehicle_ID) -> Terminator_1/1
  Antenna_Rx_4_Demux/2(GPS_pos_lat_[deg]) -> Terminator_2/1
  Antenna_Rx_4_Demux/3(GPS_pos_long_[deg]) -> Terminator_3/1
  Antenna_Rx_4_Demux/4(GPS_pos_alt_[m]) -> Terminator_4/1
  Antenna_Rx_4_Demux/5(Velocity_[m/s]) -> Terminator_5/1
  Antenna_Rx_4_Demux/6(Acceleration_[m/s2]) -> Terminator_6/1
  Antenna_Rx_4_Demux/7(Heading [deg]) -> Terminator_7/1
  Antenna_Rx_4_Demux/8(warning_signal_id) -> Terminator_8/1
  Antenna_Rx_4_Demux/9(maneuvre_id) -> Terminator_9/1
  Antenna_Rx_4_Demux/10(vehicle_type) -> Terminator_10/1
  Antenna_Rx_4_Demux/11(brakingcapacity) -> Terminator_11/1
  Antenna_Rx_4_Demux/12(vehicle_size) -> Terminator_12/1
  Ground_1/1 -> Antenna_Tx_22_Mux/1(warning_signal_id)
  Ground_2/1 -> Antenna_Tx_22_Mux/2(maneuvre_id)
  Ground_3/1 -> Antenna_Tx_22_Mux/3(vehicle_type)
  Ground_4/1 -> Antenna_Tx_22_Mux/4(brakingcapacity)
  Ground_5/1 -> Antenna_Tx_22_Mux/5(vehicle_size)
  SELF_Demux/4(rotx [deg]) -> Terminator_13/1
  SELF_Demux/5(roty [deg]) -> Terminator_14/1
  SELF_Demux/11(heading [deg]) -> Terminator_15/1

== system V2x_TC_TrafficLights_cs/Fiat_Bravo_1 ==
  SELF_Fiat_Bravo_1 : Inport  Tag=SELFInport
  Antenna_Rx_2 : Inport  Tag=AntennaRInport  Port=2
  Antenna_Rx_2_BusSelector : BusSelector  Tag=BusAntennaR  OutputSignals=warning,messageID,actorID,actorType,publicActorType,lightBarInUse,sireneInUse,velocity,acceleration,yawRate
  Antenna_Rx_2_Demux : Subsystem  Tag=DemuxAntennaR  mask(PreScan Demux Block:){Channel=1}
      settings: DeleteFcn=PreScanBlockDeleteFcn;
      mask Display:
      | fprintf('Channel: %d',Channel)
      | port_label('output', 1, 'Vehicle_ID')
      | port_label('output', 2, 'GPS Pos Lat [deg]')
      | port_label('output', 3, 'GPS Pos Long [deg]')
      | port_label('output', 4, 'GPS Pos Alt [m]')
      | port_label('output', 5, 'Velocity [m/s]')
      | port_label('output', 6, 'Acceleration [m/s2]')
      | port_label('output', 7, 'Heading [deg]')
      | port_label('output', 8, 'Warning signal ID')
      | port_label('output', 9, 'Maneuvre ID')
      | port_label('output', 10, 'Vehicle Type')
      | port_label('output', 11, 'Braking Capacity')
      | port_label('output', 12, 'Vehicle Size')
      | port_label('output', 13, 'Spare')
  Antenna_Tx_20_BusAssignment : BusAssignment  Tag=BusAntennaT  AssignedSignals=warning,messageID,actorID,actorType,publicActorType,lightBarInUse,sireneInUse,velocity,acceleration,yawRate,turnAdvice,trafficLightPriority,GPS_lat,GPS_long,GPS_alt,rotZ
  Antenna_Tx_20_Mux : Subsystem  Tag=MuxAntennaT  mask(PreScan Mux Block:){Channel=1, TimeStep=1/100}
      mask prompts: TimeStep "Time Step [s]"
      settings: DeleteFcn=PreScanBlockDeleteFcn;
      mask Display:
      | fprintf('Channel: %d',Channel)
      | port_label('input', 1, 'Warning signal ID')
      | port_label('input', 2, 'Maneuvre ID')
      | port_label('input', 3, 'Vehicle Type')
      | port_label('input', 4, 'Braking Capacity')
      | port_label('input', 5, 'Vehicle Size')
      | port_label('input', 6, 'spare')
  Constant : Constant  Value=0
  Ground_{1..11} (11 alike) : Ground
  SELF_Demux : Subsystem  Tag=DemuxSELF
      settings: DeleteFcn=PreScanBlockDeleteFcn;
      mask Type: PreScan Demux Block:
      mask Display:
      | port_label('output',1,'x [m]')
      | port_label('output',2,'y [m]')
      | port_label('output',3,'z [m]','texmode','on')
      | port_label('output',4,'Rotx [deg]','texmode','on')
      | port_label('output',5,'Roty [deg]','texmode','on')
      | port_label('output',6,'Rotz [deg]','texmode','on')
      | port_label('output',7,'GPS lat [deg]','texmode','on')
      | port_label('output',8,'GPS long [deg]','texmode','on')
      | port_label('output',9,'GPS alt [m]','texmode','on')
      | port_label('output',10,'Velocity [m/s]')
      | port_label('output',11,'Heading [deg]')
      | port_label('output',12,'Yaw rate [deg/s]')
  Terminator_{1..15,18..27} (25 alike) : Terminator
  Trajectories : Subsystem  Tag=Trajectories
      settings: DeleteFcn=PreScanBlockDeleteFcn;
      mask Type: PreScan Source Block: Trajectories
      mask Description:
      | The Trajectory block contains the trajectories defined in the PreScan Experiment Editor. The output of the trajectory block is the (vehicle) State.
      | 
      | For more information, please click Help.
      mask Help: eval('openHelp(312)')
      mask Display: image(imread('Trajectory.png'));
  Unit Delay1 : UnitDelay  SampleTime=-1
  Unit Delay2 : UnitDelay  SampleTime=-1
  VISUWheels_Mux : Subsystem  Tag=MuxWheels  mask(PreScan Mux Block:){TimeStep=1/100}
      mask prompts: TimeStep "Auto Assigned: Time Step [s]"
      settings: DeleteFcn=PreScanBlockDeleteFcn;
  vehicle CAM generator : Subsystem
  STATE_Fiat_Bravo_1 : Outport  Tag=StateOutport
  Antenna_Tx_20 : Outport  Tag=AntennaTOutport  Port=2
  VISUWheels_Fiat_Bravo_1 : Outport  Tag=VisuWheelsOutport  Port=3  SampleTime=1/100
  wires:
  Constant/1 -> vehicle CAM generator/10(turnAdvice)
  [GPS_Noise] -> vehicle CAM generator/11(GPS_Noise_src)
  [MUR_Noise] -> vehicle CAM generator/12(MessageUpdateRate)
  SELF_Fiat_Bravo_1/1 -> SELF_Demux/1(SELF)
  Antenna_Rx_2_Demux/13(spare) -> Antenna_Rx_2_BusSelector/1
  Antenna_Rx_2/1 -> Antenna_Rx_2_Demux/1(AntennaR)
  Antenna_Tx_20_Mux/2(Out2) -> [Antenna_Tx_20]
  [Antenna_Tx_20] -> Antenna_Tx_20_BusAssignment/1
  Antenna_Tx_20_Mux/1(Out1) -> Antenna_Tx_20/1
  VISUWheels_Mux/1(Wheels) -> VISUWheels_Fiat_Bravo_1/1
  Trajectories/1(State) -> STATE_Fiat_Bravo_1/1
  Antenna_Rx_2_BusSelector/10 -> Terminator_27/1  "<yawRate>"
  Antenna_Rx_2_BusSelector/9 -> Terminator_26/1  "<acceleration>"
  Antenna_Rx_2_BusSelector/8 -> Terminator_25/1  "<velocity>"
  Antenna_Rx_2_BusSelector/7 -> Terminator_24/1  "<sireneInUse>"
  Antenna_Rx_2_BusSelector/6 -> Terminator_23/1  "<lightBarInUse>"
  Antenna_Rx_2_BusSelector/5 -> Terminator_22/1  "<publicActorType>"
  Antenna_Rx_2_BusSelector/4 -> Terminator_21/1  "<actorType>"
  Antenna_Rx_2_BusSelector/3 -> Terminator_20/1  "<actorID>"
  Antenna_Rx_2_BusSelector/2 -> Terminator_19/1  "<messageID>"
  Antenna_Rx_2_BusSelector/1 -> Terminator_18/1  "<warning>"
  vehicle CAM generator/16(CAM_rotZ) -> Antenna_Tx_20_BusAssignment/17
  vehicle CAM generator/15(CAM_GPSalt) -> Antenna_Tx_20_BusAssignment/16
  vehicle CAM generator/14(CAM_GPSlong) -> Antenna_Tx_20_BusAssignment/15
  vehicle CAM generator/13(CAM_GPSlat) -> Antenna_Tx_20_BusAssignment/14
  vehicle CAM generator/12(CAM_trafficLightPriority) -> Antenna_Tx_20_BusAssignment/13
  vehicle CAM generator/11(CAM_turnAdvice) -> Antenna_Tx_20_BusAssignment/12
  SELF_Demux/12(yaw rate [deg/s]) -> vehicle CAM generator/9(yawRate)
  SELF_Demux/10(velocity [m/s]) -> vehicle CAM generator/8(velocity [m/s]), Unit Delay2/1
  SELF_Demux/9(GPS alt [m]) -> vehicle CAM generator/7(GPS_alt)
  SELF_Demux/8(GPS long [deg]) -> vehicle CAM generator/6(GPS_long)
  SELF_Demux/7(GPS lat [deg]) -> vehicle CAM generator/5(GPS_lat)
  SELF_Demux/6(rotz [deg]) -> vehicle CAM generator/4(rotZ [deg])
  SELF_Demux/3(z [m]) -> vehicle CAM generator/3(z [m])
  SELF_Demux/2(y [m]) -> vehicle CAM generator/2(y [m])
  SELF_Demux/1(x [m]) -> vehicle CAM generator/1(x [m])
  vehicle CAM generator/1(CAM_warning) -> Antenna_Tx_20_BusAssignment/2
  vehicle CAM generator/2(CAM_messageID) -> Antenna_Tx_20_BusAssignment/3
  vehicle CAM generator/3(CAM_actorID) -> Antenna_Tx_20_BusAssignment/4
  vehicle CAM generator/4(CAM_actorType) -> Antenna_Tx_20_BusAssignment/5
  vehicle CAM generator/5(CAM_publicActorType) -> Antenna_Tx_20_BusAssignment/6
  vehicle CAM generator/6(CAM_lightBarInUse) -> Antenna_Tx_20_BusAssignment/7
  vehicle CAM generator/7(CAM_sireneInUse) -> Antenna_Tx_20_BusAssignment/8
  vehicle CAM generator/8(CAM_velocity) -> Antenna_Tx_20_BusAssignment/9
  vehicle CAM generator/9(CAM_acceleration) -> Antenna_Tx_20_BusAssignment/10
  vehicle CAM generator/10(CAM_yawRate) -> Antenna_Tx_20_BusAssignment/11
  Unit Delay1/1 -> Antenna_Tx_20_Mux/6(spare)
  Unit Delay2/1 -> VISUWheels_Mux/3(Velocity [m/s])
  Antenna_Tx_20_BusAssignment/1 -> Unit Delay1/1
  Antenna_Rx_2_Demux/1(Vehicle_ID) -> Terminator_1/1
  Antenna_Rx_2_Demux/2(GPS_pos_lat_[deg]) -> Terminator_2/1
  Antenna_Rx_2_Demux/3(GPS_pos_long_[deg]) -> Terminator_3/1
  Antenna_Rx_2_Demux/4(GPS_pos_alt_[m]) -> Terminator_4/1
  Antenna_Rx_2_Demux/5(Velocity_[m/s]) -> Terminator_5/1
  Antenna_Rx_2_Demux/6(Acceleration_[m/s2]) -> Terminator_6/1
  Antenna_Rx_2_Demux/7(Heading [deg]) -> Terminator_7/1
  Antenna_Rx_2_Demux/8(warning_signal_id) -> Terminator_8/1
  Antenna_Rx_2_Demux/9(maneuvre_id) -> Terminator_9/1
  Antenna_Rx_2_Demux/10(vehicle_type) -> Terminator_10/1
  Antenna_Rx_2_Demux/11(brakingcapacity) -> Terminator_11/1
  Antenna_Rx_2_Demux/12(vehicle_size) -> Terminator_12/1
  Ground_1/1 -> Antenna_Tx_20_Mux/1(warning_signal_id)
  Ground_2/1 -> Antenna_Tx_20_Mux/2(maneuvre_id)
  Ground_3/1 -> Antenna_Tx_20_Mux/3(vehicle_type)
  Ground_4/1 -> Antenna_Tx_20_Mux/4(brakingcapacity)
  Ground_5/1 -> Antenna_Tx_20_Mux/5(vehicle_size)
  SELF_Demux/4(rotx [deg]) -> Terminator_13/1
  SELF_Demux/5(roty [deg]) -> Terminator_14/1
  SELF_Demux/11(heading [deg]) -> Terminator_15/1
  Ground_6/1 -> VISUWheels_Mux/1(Steer Angle Front [deg])
  Ground_7/1 -> VISUWheels_Mux/2(Steer Angle Rear [deg])
  Ground_8/1 -> VISUWheels_Mux/4(zDisp FL [m])
  Ground_9/1 -> VISUWheels_Mux/5(zDisp FR [m])
  Ground_10/1 -> VISUWheels_Mux/6(zDisp RL [m])
  Ground_11/1 -> VISUWheels_Mux/7(zDisp RR [m])
